simulink model "MX_Gateway"  (Simulink 9.0) (continued)
== stateflow chart MX_Gtwy_Control/System/Aux_Enable/Aux_Control == (continued)
          state Wait
            en:
            aux_Flow = AUX_RELEASE_WAIT;
            cmd_AuxRelease = INACTIVE;
          state Ack
            en:
            aux_Flow = AUX_RELEASE_ACK;
          state Release_Base
            en:
            aux_Flow = AUX_RELEASE_IN_PROGRESS;
            cmd_AuxRelease = BASE_ACTIVE;
          state Release_Rod
            en:
            cmd_AuxRelease = ROD_ACTIVE;
          state Confirm
            en:
            aux_Flow = AUX_RELEASE_CONFIRM;
            cmd_AuxRelease = INACTIVE;
          (default) -> Aux_Enable_State.PHOENIX_K2.Functional.Aux_Relief_G5.Wait
          Aux_Enable_State.PHOENIX_K2.Functional.Aux_Relief_G5.Wait -> Ack : [button_Aux]
          Ack -> Aux_Enable_State.PHOENIX_K2.Functional.Aux_Relief_G5.Wait [order 2] : [button_AuxReleaseBack]
          Aux_Enable_State.PHOENIX_K2.Functional.Aux_Relief_G5.Confirm -> Aux_Enable_State.PHOENIX_K2.Functional.Aux_Relief_G5.Wait : [after(CD_AUX_CONFIRM_TIME, tick)...
              | || button_AuxReleaseExit]
          Ack -> Aux_Enable_State.PHOENIX_K2.Functional.Aux_Relief_G5.Release_Base [order 1] : [button_AuxReleaseAck]
          Aux_Enable_State.PHOENIX_K2.Functional.Aux_Relief_G5.Release_Base -> Aux_Enable_State.PHOENIX_K2.Functional.Aux_Relief_G5.Release_Rod : [after(CD_AUX_RELEASE_TIME, tick)]
          Aux_Enable_State.PHOENIX_K2.Functional.Aux_Relief_G5.Release_Rod -> Aux_Enable_State.PHOENIX_K2.Functional.Aux_Relief_G5.Confirm : [after(CD_AUX_RELEASE_TIME, tick)]
        state Aux_Relief_G55 [commented out]
          state Wait [commented out]
            en:
            state_AuxRelease = ACTIVE;
            cmd_AuxRelease = INACTIVE;
          state Release_Base [commented out]
            en:
            cmd_AuxRelease = BASE_ACTIVE;
          state Release_Rod [commented out]
            en:
            cmd_AuxRelease = ROD_ACTIVE;
          state Confirm [commented out]
            en:
            cmd_AuxRelease = INACTIVE;
          (default) -> Aux_Enable_State.PHOENIX_K2.Functional.Aux_Relief_G55.Wait [commented out]
          Aux_Enable_State.PHOENIX_K2.Functional.Aux_Relief_G55.Wait -> Aux_Enable_State.PHOENIX_K2.Functional.Aux_Relief_G55.Release_Base [commented out] : [dlx_G55_AuxReleaseStatus == ACTIVE]
          Aux_Enable_State.PHOENIX_K2.Functional.Aux_Relief_G55.Confirm -> Aux_Enable_State.PHOENIX_K2.Functional.Aux_Relief_G55.Wait [commented out] : [dlx_G55_AuxReleaseStatus == INACTIVE]
          Aux_Enable_State.PHOENIX_K2.Functional.Aux_Relief_G55.Release_Base -> Aux_Enable_State.PHOENIX_K2.Functional.Aux_Relief_G55.Release_Rod [commented out] : [after(CD_AUX_RELEASE_TIME, tick)]
          Aux_Enable_State.PHOENIX_K2.Functional.Aux_Relief_G55.Release_Rod -> Aux_Enable_State.PHOENIX_K2.Functional.Aux_Relief_G55.Confirm [commented out] : [after(CD_AUX_RELEASE_TIME, tick)]
        (default) -> Aux_Enable_State.PHOENIX_K2.Functional.Inactive
        Aux_Enable_State.PHOENIX_K2.Functional.Inactive_Fault -> Aux_Enable_State.PHOENIX_K2.Functional.Enabled.Off : [state_AuxPot != OORH...
            |  && state_AuxPot != OORL...
            |  && state_System == SYS_NORMAL]
        Aux_Enable_State.PHOENIX_K2.Functional.Inactive -> Aux_Enable_State.PHOENIX_K2.Functional.Enabled [order 1] : [state_Engine != ENGINE_CRANKING...
            |  && (state_Engine == ENGINE_RUNNING...
            |     || CD_AUX_RELEASE_FUNCTION == NON_FUNCTIONAL...
            |     || (!ee_DeluxeG5...
            |          && CD_DELUXE_GEN5_5_FUNCTION == NON_FUNCTIONAL))...
            |  && state_System == SYS_NORMAL...
            |  && state_Console == ACTIVE...
            |  && flag_InitEERead]
        Aux_Enable_State.PHOENIX_K2.Functional.Enabled -> Aux_Enable_State.PHOENIX_K2.Functional.Inactive_Fault [order 2] : [state_AuxPot == OORH...
            |  || state_AuxPot == OORL]
        Aux_Enable_State.PHOENIX_K2.Functional.Enabled -> Aux_Enable_State.PHOENIX_K2.Functional.Inactive [order 1] : [state_Engine == ENGINE_CRANKING...
            |  || state_System != SYS_NORMAL...
            |  || state_Console != ACTIVE]
        Aux_Enable_State.PHOENIX_K2.Functional.Inactive_Out_Of_Neutral -> Aux_Enable_State.PHOENIX_K2.Functional.Inactive_Fault [order 2] : [state_AuxPot == OORH...
            |  || state_AuxPot == OORL]
        Aux_Enable_State.PHOENIX_K2.Functional.Enabled -> Aux_Enable_State.PHOENIX_K2.Functional.Inactive_Out_Of_Neutral [order 3] : [state_AuxPot == NOT_IN_NEUTRAL]
        (junction 2117) -> Aux_Enable_State.PHOENIX_K2.Functional.Enabled
        (junction 2116) -> (junction 2115) [order 1] : [(aux_DebounceButton...
            | || aux_DetentHold)...
            | && aux_Flow != AUX_FLOW_INACTIVE]
        Aux_Enable_State.PHOENIX_K2.Functional.Inactive_Out_Of_Neutral -> (junction 2116) [order 3] : [state_AuxPot != NOT_IN_NEUTRAL]
        (junction 2115) -> (junction 2117) : {state_Buzzer = SINGLE_SHORT_BEEP;}
        (junction 2116) -> (junction 2118) [order 2]
        (junction 2118) -> (junction 2117)
        Aux_Enable_State.PHOENIX_K2.Functional.Inactive_Out_Of_Neutral -> Aux_Enable_State.PHOENIX_K2.Functional.Inactive [order 1] : [state_Console != ACTIVE]
        Aux_Enable_State.PHOENIX_K2.Functional.Inactive -> Aux_Relief_G5 [order 2] : [(CD_AUX_RELEASE_FUNCTION != NON_FUNCTIONAL)...
            | && ((state_Engine == ENGINE_NOT_RUNNING) || (state_Engine == ENGINE_INITIALIZE))...
            | && (state_EngineSpeed != NO_SIGNAL)...
            | && (state_System == SYS_NORMAL)...
            | && (ee_DeluxeG5) && flag_InitEERead...
            | && (CD_DELUXE_GEN5_5_FUNCTION == NON_FUNCTIONAL)]
        Aux_Relief_G5 -> Aux_Enable_State.PHOENIX_K2.Functional.Inactive : [((state_Engine != ENGINE_NOT_RUNNING) && (state_Engine != ENGINE_INITIALIZE))...
            |  || (state_EngineSpeed == NO_SIGNAL)...
            |  || (state_System != SYS_NORMAL)]
        Aux_Enable_State.PHOENIX_K2.Functional.Inactive -> Aux_Relief_G55 [commented out] : [(CD_AUX_RELEASE_FUNCTION != NON_FUNCTIONAL)...
            | && ((state_Engine == ENGINE_NOT_RUNNING) || (state_Engine == ENGINE_INITIALIZE))...
            | && (state_EngineSpeed != NO_SIGNAL)...
            | && (state_System == SYS_NORMAL)...
            | && (!ee_DeluxeG5)...
            | && (CD_DELUXE_GEN5_5_FUNCTION != NON_FUNCTIONAL)]
        Aux_Relief_G55 -> Aux_Enable_State.PHOENIX_K2.Functional.Inactive [commented out] : [((state_Engine != ENGINE_NOT_RUNNING) && (state_Engine != ENGINE_INITIALIZE))...
            |  || (state_System != SYS_NORMAL)]
      state Aux_Enable_State_ST
        state Inactive_Latched
          en:
          state_AuxEnable = INACTIVE;
        state Inactive_Temp
          en:
          state_AuxEnable = INACTIVE;
        state Standard_Flow
          en:
          aux_Flow = AUX_FLOW_STANDARD;
          state_AuxEnable = ACTIVE_MODE_ONE;
          ee_AuxFlow = AUX_FLOW_INACTIVE;
          matlab_UpdateEEVar();
        (default) -> Aux_Enable_State.PHOENIX_K2.Aux_Enable_State_ST.Standard_Flow
        Aux_Enable_State.PHOENIX_K2.Aux_Enable_State_ST.Standard_Flow -> Aux_Enable_State.PHOENIX_K2.Aux_Enable_State_ST.Inactive_Temp [order 1] : [state_Engine == ENGINE_CRANKING]
        Aux_Enable_State.PHOENIX_K2.Aux_Enable_State_ST.Standard_Flow -> Aux_Enable_State.PHOENIX_K2.Aux_Enable_State_ST.Inactive_Latched [order 2] : [state_AuxPot == OORH...
            |  || state_AuxPot == OORL...
            |  || state_Console == OORL...
            |  || state_Console == OORH...
            |  || state_AuxPot == NOT_IN_NEUTRAL...
            |  || state_System != SYS_NORMAL]
        Aux_Enable_State.PHOENIX_K2.Aux_Enable_State_ST.Inactive_Temp -> Aux_Enable_State.PHOENIX_K2.Aux_Enable_State_ST.Standard_Flow : [state_Engine != ENGINE_CRANKING]
        Aux_Enable_State.PHOENIX_K2.Aux_Enable_State_ST.Standard_Flow -> Aux_Enable_State.PHOENIX_K2.Aux_Enable_State_ST.Inactive_Latched [order 3] : disable_aux
      state Aux_Enable_State_ACD
        state Inactive_Latched
          en:
          state_AuxEnable = INACTIVE;
        state Inactive_Temp
          en:
          state_AuxEnable = INACTIVE;
        state Standard_Flow
          en:
          aux_Flow = AUX_FLOW_STANDARD;
          state_AuxEnable = ACTIVE_MODE_ONE;
          ee_AuxFlow = AUX_FLOW_INACTIVE;
          matlab_UpdateEEVar();
        (default) -> Aux_Enable_State.PHOENIX_K2.Aux_Enable_State_ACD.Standard_Flow
        Aux_Enable_State.PHOENIX_K2.Aux_Enable_State_ACD.Standard_Flow -> Aux_Enable_State.PHOENIX_K2.Aux_Enable_State_ACD.Inactive_Temp [order 1] : [state_Engine == ENGINE_CRANKING]
        Aux_Enable_State.PHOENIX_K2.Aux_Enable_State_ACD.Standard_Flow -> Aux_Enable_State.PHOENIX_K2.Aux_Enable_State_ACD.Inactive_Latched [order 2] : [state_AuxPot == OORH...
            |  || state_AuxPot == OORL...
            |  || state_Console == OORL...
            |  || state_Console == OORH...
            |  || state_AuxPot == NOT_IN_NEUTRAL...
            |  || state_System != SYS_NORMAL]
        Aux_Enable_State.PHOENIX_K2.Aux_Enable_State_ACD.Standard_Flow -> Aux_Enable_State.PHOENIX_K2.Aux_Enable_State_ACD.Inactive_Latched [order 3] : disable_aux
        Aux_Enable_State.PHOENIX_K2.Aux_Enable_State_ACD.Inactive_Temp -> Aux_Enable_State.PHOENIX_K2.Aux_Enable_State_ACD.Standard_Flow : [state_Engine != ENGINE_CRANKING]
      (default) -> Aux_Enable_State.PHOENIX_K2.Functional
      Aux_Enable_State.PHOENIX_K2.Functional -> Aux_Enable_State.PHOENIX_K2.Aux_Enable_State_ST [order 1] : [(can_AuxEnable_ST == ACTIVE)...
          | && (state_ST_CAN == NORMAL)...
          | && (CD_STAUX_FUNCTION != NON_FUNCTIONAL)]
      Aux_Enable_State.PHOENIX_K2.Aux_Enable_State_ST -> Aux_Enable_State.PHOENIX_K2.Functional : [(can_AuxEnable_ST == INACTIVE)...
          | || (state_ST_CAN != NORMAL)]
      Aux_Enable_State.PHOENIX_K2.Functional -> Aux_Enable_State.PHOENIX_K2.Aux_Enable_State_ACD [order 2] : [(can_AuxEnable_ACD == ACTIVE)...
          | && (state_ACD_CAN == NORMAL)...
          | && (CD_RACD_FUNCTION != NON_FUNCTIONAL)]
      Aux_Enable_State.PHOENIX_K2.Aux_Enable_State_ACD -> Aux_Enable_State.PHOENIX_K2.Functional : [(can_AuxEnable_ACD == INACTIVE)...
          | || (state_ACD_CAN != NORMAL)]
    state SIRIUS2
      state Functional
        state Enabled
          state Off
            en:
            aux_Flow = AUX_FLOW_INACTIVE;
            ee_AuxFlow = AUX_FLOW_INACTIVE;
            state_AuxEnable = INACTIVE;
            matlab_UpdateEEVar();
          state Active
            state Low_Flow
              en:
              aux_Flow = AUX_FLOW_LOW;
              ee_AuxFlow = AUX_FLOW_LOW;
              state_AuxEnable = ACTIVE_MODE_ONE;
              matlab_UpdateEEVar();
            state Medium_Flow
              en:
              aux_Flow = AUX_FLOW_MEDIUM;
              ee_AuxFlow = AUX_FLOW_MEDIUM;
              state_AuxEnable = ACTIVE_MODE_ONE;
              matlab_UpdateEEVar();
            state Standard_Flow
              en:
              aux_Flow = AUX_FLOW_STANDARD;
              ee_AuxFlow = AUX_FLOW_STANDARD;
              state_AuxEnable = ACTIVE_MODE_ONE;
              matlab_UpdateEEVar();
            Aux_Enable_State.SIRIUS2.Functional.Enabled.Active.Medium_Flow -> Aux_Enable_State.SIRIUS2.Functional.Enabled.Active.Low_Flow : [aux_DebounceButton]
                | {state_Buzzer = SINGLE_SHORT_BEEP;}
            Aux_Enable_State.SIRIUS2.Functional.Enabled.Active.Low_Flow -> Aux_Enable_State.SIRIUS2.Functional.Enabled.Active.Standard_Flow [order 1] : [aux_DebounceButton...
                | && state_DetentEnable == ACTIVE]
                | {state_Buzzer = SINGLE_SHORT_BEEP;}
            Aux_Enable_State.SIRIUS2.Functional.Enabled.Active.Standard_Flow -> Aux_Enable_State.SIRIUS2.Functional.Enabled.Active.Medium_Flow : [aux_DebounceButton]
                | {state_Buzzer = SINGLE_SHORT_BEEP;}
          (junction 2206) -> Aux_Enable_State.SIRIUS2.Functional.Enabled.Off [order 1] : [ee_AuxFlow == AUX_FLOW_INACTIVE]
          (default) -> (junction 2206)
          (junction 2206) -> (junction 2207) [order 2]
          Aux_Enable_State.SIRIUS2.Functional.Enabled.Active.Low_Flow -> Aux_Enable_State.SIRIUS2.Functional.Enabled.Off [order 2] : [aux_DebounceButton...
              | && state_DetentEnable != ACTIVE]
              | {state_Buzzer = SINGLE_SHORT_BEEP;}
          Aux_Enable_State.SIRIUS2.Functional.Enabled.Off -> Aux_Enable_State.SIRIUS2.Functional.Enabled.Active.Standard_Flow : [aux_DebounceButton...
              | || aux_DetentHold]
              | {state_Buzzer = SINGLE_SHORT_BEEP;}
          Aux_Enable_State.SIRIUS2.Functional.Enabled.Active -> Aux_Enable_State.SIRIUS2.Functional.Enabled.Off : disable_aux
          (junction 2207) -> Aux_Enable_State.SIRIUS2.Functional.Enabled.Active.Low_Flow [order 1] : [ee_AuxFlow == AUX_FLOW_LOW]
          (junction 2207) -> (junction 2208) [order 2]
          (junction 2208) -> Aux_Enable_State.SIRIUS2.Functional.Enabled.Active.Medium_Flow [order 1] : [ee_AuxFlow == AUX_FLOW_MEDIUM]
          (junction 2208) -> (junction 2209) [order 2]
          (junction 2209) -> Aux_Enable_State.SIRIUS2.Functional.Enabled.Active.Standard_Flow
        state Inactive
          en:
          state_AuxEnable = INACTIVE;
          cmd_AuxRelease = INACTIVE;
          aux_Flow = AUX_FLOW_INACTIVE;
        state Inactive_Fault
          en:
          state_AuxEnable = INACTIVE;
          aux_Flow = AUX_FLOW_INACTIVE;
        state Inactive_Out_Of_Neutral
          en:
          state_AuxEnable = INACTIVE;
          aux_Flow = AUX_FLOW_INACTIVE;
        (default) -> Aux_Enable_State.SIRIUS2.Functional.Inactive
        Aux_Enable_State.SIRIUS2.Functional.Inactive_Fault -> Aux_Enable_State.SIRIUS2.Functional.Enabled.Off : [state_AuxPot != OORH...
            |  && state_AuxPot != OORL...
            |  && state_System == SYS_NORMAL]
        Aux_Enable_State.SIRIUS2.Functional.Inactive -> Aux_Enable_State.SIRIUS2.Functional.Enabled : [state_Engine != ENGINE_CRANKING...
            |  && state_System == SYS_NORMAL...
            |  && state_Console == ACTIVE...
            |  && flag_InitEERead]
        Aux_Enable_State.SIRIUS2.Functional.Enabled -> Aux_Enable_State.SIRIUS2.Functional.Inactive_Fault [order 2] : [state_AuxPot == OORH...
            |  || state_AuxPot == OORL]
        Aux_Enable_State.SIRIUS2.Functional.Enabled -> Aux_Enable_State.SIRIUS2.Functional.Inactive [order 1] : [state_Engine == ENGINE_CRANKING...
            |  || state_System != SYS_NORMAL...
            |  || state_Console != ACTIVE]
        Aux_Enable_State.SIRIUS2.Functional.Inactive_Out_Of_Neutral -> Aux_Enable_State.SIRIUS2.Functional.Inactive_Fault [order 2] : [state_AuxPot == OORH...
            |  || state_AuxPot == OORL]
        Aux_Enable_State.SIRIUS2.Functional.Enabled -> Aux_Enable_State.SIRIUS2.Functional.Inactive_Out_Of_Neutral [order 3] : [state_AuxPot == NOT_IN_NEUTRAL]
        (junction 2190) -> Aux_Enable_State.SIRIUS2.Functional.Enabled
        (junction 2189) -> (junction 2188) [order 1] : [(aux_DebounceButton...
            | || aux_DetentHold)...
            | && aux_Flow != AUX_FLOW_INACTIVE]
        Aux_Enable_State.SIRIUS2.Functional.Inactive_Out_Of_Neutral -> (junction 2189) [order 3] : [state_AuxPot != NOT_IN_NEUTRAL]
        (junction 2188) -> (junction 2190) : {state_Buzzer = SINGLE_SHORT_BEEP;}
        (junction 2189) -> (junction 2191) [order 2]
        (junction 2191) -> (junction 2190)
        Aux_Enable_State.SIRIUS2.Functional.Inactive_Out_Of_Neutral -> Aux_Enable_State.SIRIUS2.Functional.Inactive [order 1] : [state_Console != ACTIVE]
      state Aux_Enable_State_ST
        state Inactive_Latched
          en:
          state_AuxEnable = INACTIVE;
        state Inactive_Temp
          en:
          state_AuxEnable = INACTIVE;
        state Standard_Flow
          en:
          aux_Flow = AUX_FLOW_STANDARD;
          state_AuxEnable = ACTIVE_MODE_ONE;
          ee_AuxFlow = AUX_FLOW_INACTIVE;
          matlab_UpdateEEVar();
        (default) -> Aux_Enable_State.SIRIUS2.Aux_Enable_State_ST.Standard_Flow
        Aux_Enable_State.SIRIUS2.Aux_Enable_State_ST.Standard_Flow -> Aux_Enable_State.SIRIUS2.Aux_Enable_State_ST.Inactive_Temp [order 1] : [state_Engine == ENGINE_CRANKING]
        Aux_Enable_State.SIRIUS2.Aux_Enable_State_ST.Standard_Flow -> Aux_Enable_State.SIRIUS2.Aux_Enable_State_ST.Inactive_Latched [order 2] : [state_AuxPot == OORH...
            |  || state_AuxPot == OORL...
            |  || state_Console == OORL...
            |  || state_Console == OORH...
            |  || state_AuxPot == NOT_IN_NEUTRAL...
            |  || state_System != SYS_NORMAL]
        Aux_Enable_State.SIRIUS2.Aux_Enable_State_ST.Inactive_Temp -> Aux_Enable_State.SIRIUS2.Aux_Enable_State_ST.Standard_Flow : [state_Engine != ENGINE_CRANKING]
        Aux_Enable_State.SIRIUS2.Aux_Enable_State_ST.Standard_Flow -> Aux_Enable_State.SIRIUS2.Aux_Enable_State_ST.Inactive_Latched [order 3] : disable_aux
      state Aux_Enable_State_ACD
        state Inactive_Latched
          en:
          state_AuxEnable = INACTIVE;
        state Inactive_Temp
          en:
          state_AuxEnable = INACTIVE;
        state Standard_Flow
          en:
          aux_Flow = AUX_FLOW_STANDARD;
          state_AuxEnable = ACTIVE_MODE_ONE;
          ee_AuxFlow = AUX_FLOW_INACTIVE;
          matlab_UpdateEEVar();
        (default) -> Aux_Enable_State.SIRIUS2.Aux_Enable_State_ACD.Standard_Flow
        Aux_Enable_State.SIRIUS2.Aux_Enable_State_ACD.Standard_Flow -> Aux_Enable_State.SIRIUS2.Aux_Enable_State_ACD.Inactive_Temp [order 1] : [state_Engine == ENGINE_CRANKING]
        Aux_Enable_State.SIRIUS2.Aux_Enable_State_ACD.Standard_Flow -> Aux_Enable_State.SIRIUS2.Aux_Enable_State_ACD.Inactive_Latched [order 2] : [state_AuxPot == OORH...
            |  || state_AuxPot == OORL...
            |  || state_Console == OORL...
            |  || state_Console == OORH...
            |  || state_AuxPot == NOT_IN_NEUTRAL...
            |  || state_System != SYS_NORMAL]
        Aux_Enable_State.SIRIUS2.Aux_Enable_State_ACD.Standard_Flow -> Aux_Enable_State.SIRIUS2.Aux_Enable_State_ACD.Inactive_Latched [order 3] : disable_aux
        Aux_Enable_State.SIRIUS2.Aux_Enable_State_ACD.Inactive_Temp -> Aux_Enable_State.SIRIUS2.Aux_Enable_State_ACD.Standard_Flow : [state_Engine != ENGINE_CRANKING]
      (default) -> Aux_Enable_State.SIRIUS2.Functional
      Aux_Enable_State.SIRIUS2.Functional -> Aux_Enable_State.SIRIUS2.Aux_Enable_State_ST [order 1] : [(can_AuxEnable_ST == ACTIVE)...
          | && (state_ST_CAN == NORMAL)...
          | && (CD_STAUX_FUNCTION != NON_FUNCTIONAL)]
      Aux_Enable_State.SIRIUS2.Aux_Enable_State_ST -> Aux_Enable_State.SIRIUS2.Functional : [(can_AuxEnable_ST == INACTIVE)...
          | || (state_ST_CAN != NORMAL)]
      Aux_Enable_State.SIRIUS2.Functional -> Aux_Enable_State.SIRIUS2.Aux_Enable_State_ACD [order 2] : [(can_AuxEnable_ACD == ACTIVE)...
          | && (state_ACD_CAN == NORMAL)...
          | && (CD_RACD_FUNCTION != NON_FUNCTIONAL)]
      Aux_Enable_State.SIRIUS2.Aux_Enable_State_ACD -> Aux_Enable_State.SIRIUS2.Functional : [(can_AuxEnable_ACD == INACTIVE)...
          | || (state_ACD_CAN != NORMAL)]
    state SIRIUS2_HIFLOW
      state Functional
        state Enabled
          state Off
            en:
            aux_Flow = AUX_FLOW_INACTIVE;
            ee_AuxFlow = AUX_FLOW_INACTIVE;
            state_AuxEnable = INACTIVE;
            matlab_UpdateEEVar();
          state Active
            state Low_Flow
              en:
              aux_Flow = AUX_FLOW_LOW;
              ee_AuxFlow = AUX_FLOW_LOW;
              state_AuxEnable = ACTIVE_MODE_ONE;
              matlab_UpdateEEVar();
            state Medium_Flow
              en:
              aux_Flow = AUX_FLOW_MEDIUM;
              ee_AuxFlow = AUX_FLOW_MEDIUM;
              state_AuxEnable = ACTIVE_MODE_ONE;
              matlab_UpdateEEVar();
            state Standard_Flow
              en:
              aux_Flow = AUX_FLOW_STANDARD;
              ee_AuxFlow = AUX_FLOW_STANDARD;
              state_AuxEnable = ACTIVE_MODE_ONE;
              matlab_UpdateEEVar();
            state High_Flow
              en:
              aux_Flow = AUX_FLOW_HIGH;
              ee_AuxFlow = AUX_FLOW_HIGH;
              state_AuxEnable = ACTIVE_MODE_ONE;
              matlab_UpdateEEVar();
            Aux_Enable_State.SIRIUS2_HIFLOW.Functional.Enabled.Active.Medium_Flow -> Aux_Enable_State.SIRIUS2_HIFLOW.Functional.Enabled.Active.Low_Flow : [aux_DebounceButton]
                | {state_Buzzer = SINGLE_SHORT_BEEP;}
            Aux_Enable_State.SIRIUS2_HIFLOW.Functional.Enabled.Active.Low_Flow -> High_Flow [order 1] : [aux_DebounceButton...
                | && state_DetentEnable == ACTIVE]
                | {state_Buzzer = SINGLE_SHORT_BEEP;}
            Aux_Enable_State.SIRIUS2_HIFLOW.Functional.Enabled.Active.Standard_Flow -> Aux_Enable_State.SIRIUS2_HIFLOW.Functional.Enabled.Active.Medium_Flow : [aux_DebounceButton]
                | {state_Buzzer = SINGLE_SHORT_BEEP;}
            High_Flow -> Aux_Enable_State.SIRIUS2_HIFLOW.Functional.Enabled.Active.Standard_Flow : [aux_DebounceButton]
                | {state_Buzzer = SINGLE_SHORT_BEEP;}
          (junction 2257) -> Aux_Enable_State.SIRIUS2_HIFLOW.Functional.Enabled.Off [order 1] : [ee_AuxFlow == AUX_FLOW_INACTIVE]
          (default) -> (junction 2257)
          (junction 2257) -> (junction 2258) [order 2]
          Aux_Enable_State.SIRIUS2_HIFLOW.Functional.Enabled.Active.Low_Flow -> Aux_Enable_State.SIRIUS2_HIFLOW.Functional.Enabled.Off [order 2] : [aux_DebounceButton...
              | && state_DetentEnable != ACTIVE]
              | {state_Buzzer = SINGLE_SHORT_BEEP;}
          Aux_Enable_State.SIRIUS2_HIFLOW.Functional.Enabled.Off -> High_Flow : [aux_DebounceButton...
              | || aux_DetentHold]
              | {state_Buzzer = SINGLE_SHORT_BEEP;}
          Aux_Enable_State.SIRIUS2_HIFLOW.Functional.Enabled.Active -> Aux_Enable_State.SIRIUS2_HIFLOW.Functional.Enabled.Off : disable_aux
          (junction 2258) -> Aux_Enable_State.SIRIUS2_HIFLOW.Functional.Enabled.Active.Low_Flow [order 1] : [ee_AuxFlow == AUX_FLOW_LOW]
          (junction 2258) -> (junction 2259) [order 2]
          (junction 2259) -> Aux_Enable_State.SIRIUS2_HIFLOW.Functional.Enabled.Active.Medium_Flow [order 1] : [ee_AuxFlow == AUX_FLOW_MEDIUM]
          (junction 2259) -> (junction 2260) [order 2]
          (junction 2260) -> Aux_Enable_State.SIRIUS2_HIFLOW.Functional.Enabled.Active.Standard_Flow [order 1] : [ee_AuxFlow == AUX_FLOW_STANDARD]
          (junction 2260) -> (junction 2261) [order 2]
          (junction 2261) -> High_Flow
        state Inactive
          en:
          state_AuxEnable = INACTIVE;
          cmd_AuxRelease = INACTIVE;
          aux_Flow = AUX_FLOW_INACTIVE;
        state Inactive_Fault
          en:
          state_AuxEnable = INACTIVE;
          aux_Flow = AUX_FLOW_INACTIVE;
        state Inactive_Out_Of_Neutral
          en:
          state_AuxEnable = INACTIVE;
          aux_Flow = AUX_FLOW_INACTIVE;
        (default) -> Aux_Enable_State.SIRIUS2_HIFLOW.Functional.Inactive
        Aux_Enable_State.SIRIUS2_HIFLOW.Functional.Inactive_Fault -> Aux_Enable_State.SIRIUS2_HIFLOW.Functional.Enabled.Off : [state_AuxPot != OORH...
            |  && state_AuxPot != OORL...
            |  && state_System == SYS_NORMAL]
        Aux_Enable_State.SIRIUS2_HIFLOW.Functional.Inactive -> Aux_Enable_State.SIRIUS2_HIFLOW.Functional.Enabled : [state_Engine != ENGINE_CRANKING...
            |  && state_System == SYS_NORMAL...
            |  && state_Console == ACTIVE...
            |  && flag_InitEERead]
        Aux_Enable_State.SIRIUS2_HIFLOW.Functional.Enabled -> Aux_Enable_State.SIRIUS2_HIFLOW.Functional.Inactive_Fault [order 2] : [state_AuxPot == OORH...
            |  || state_AuxPot == OORL]
        Aux_Enable_State.SIRIUS2_HIFLOW.Functional.Enabled -> Aux_Enable_State.SIRIUS2_HIFLOW.Functional.Inactive [order 1] : [state_Engine == ENGINE_CRANKING...
            |  || state_System != SYS_NORMAL...
            |  || state_Console != ACTIVE]
        Aux_Enable_State.SIRIUS2_HIFLOW.Functional.Inactive_Out_Of_Neutral -> Aux_Enable_State.SIRIUS2_HIFLOW.Functional.Inactive_Fault [order 2] : [state_AuxPot == OORH...
            |  || state_AuxPot == OORL]
        Aux_Enable_State.SIRIUS2_HIFLOW.Functional.Enabled -> Aux_Enable_State.SIRIUS2_HIFLOW.Functional.Inactive_Out_Of_Neutral [order 3] : [state_AuxPot == NOT_IN_NEUTRAL]
        (junction 2241) -> Aux_Enable_State.SIRIUS2_HIFLOW.Functional.Enabled
        (junction 2240) -> (junction 2239) [order 1] : [(aux_DebounceButton...
            | || aux_DetentHold)...
            | && aux_Flow != AUX_FLOW_INACTIVE]
        Aux_Enable_State.SIRIUS2_HIFLOW.Functional.Inactive_Out_Of_Neutral -> (junction 2240) [order 3] : [state_AuxPot != NOT_IN_NEUTRAL]
        (junction 2239) -> (junction 2241) : {state_Buzzer = SINGLE_SHORT_BEEP;}
        (junction 2240) -> (junction 2242) [order 2]
        (junction 2242) -> (junction 2241)
        Aux_Enable_State.SIRIUS2_HIFLOW.Functional.Inactive_Out_Of_Neutral -> Aux_Enable_State.SIRIUS2_HIFLOW.Functional.Inactive [order 1] : [state_Console != ACTIVE]
      state Aux_Enable_State_ST
        state Inactive_Latched
          en:
          state_AuxEnable = INACTIVE;
        state Inactive_Temp
          en:
          state_AuxEnable = INACTIVE;
        state Standard_Flow
          en:
          aux_Flow = AUX_FLOW_STANDARD;
          state_AuxEnable = ACTIVE_MODE_ONE;
          ee_AuxFlow = AUX_FLOW_INACTIVE;
          matlab_UpdateEEVar();
        (default) -> Aux_Enable_State.SIRIUS2_HIFLOW.Aux_Enable_State_ST.Standard_Flow
        Aux_Enable_State.SIRIUS2_HIFLOW.Aux_Enable_State_ST.Standard_Flow -> Aux_Enable_State.SIRIUS2_HIFLOW.Aux_Enable_State_ST.Inactive_Temp [order 1] : [state_Engine == ENGINE_CRANKING]
        Aux_Enable_State.SIRIUS2_HIFLOW.Aux_Enable_State_ST.Standard_Flow -> Aux_Enable_State.SIRIUS2_HIFLOW.Aux_Enable_State_ST.Inactive_Latched [order 2] : [state_AuxPot == OORH...
            |  || state_AuxPot == OORL...
            |  || state_Console == OORL...
            |  || state_Console == OORH...
            |  || state_AuxPot == NOT_IN_NEUTRAL...
            |  || state_System != SYS_NORMAL]
        Aux_Enable_State.SIRIUS2_HIFLOW.Aux_Enable_State_ST.Inactive_Temp -> Aux_Enable_State.SIRIUS2_HIFLOW.Aux_Enable_State_ST.Standard_Flow : [state_Engine != ENGINE_CRANKING]
        Aux_Enable_State.SIRIUS2_HIFLOW.Aux_Enable_State_ST.Standard_Flow -> Aux_Enable_State.SIRIUS2_HIFLOW.Aux_Enable_State_ST.Inactive_Latched [order 3] : disable_aux
      state Aux_Enable_State_ACD
        state Inactive_Latched
          en:
          state_AuxEnable = INACTIVE;
        state Inactive_Temp
          en:
          state_AuxEnable = INACTIVE;
        state Standard_Flow
          en:
          aux_Flow = AUX_FLOW_STANDARD;
          state_AuxEnable = ACTIVE_MODE_ONE;
          ee_AuxFlow = AUX_FLOW_INACTIVE;
          matlab_UpdateEEVar();
        (default) -> Aux_Enable_State.SIRIUS2_HIFLOW.Aux_Enable_State_ACD.Standard_Flow
        Aux_Enable_State.SIRIUS2_HIFLOW.Aux_Enable_State_ACD.Standard_Flow -> Aux_Enable_State.SIRIUS2_HIFLOW.Aux_Enable_State_ACD.Inactive_Temp [order 1] : [state_Engine == ENGINE_CRANKING]
        Aux_Enable_State.SIRIUS2_HIFLOW.Aux_Enable_State_ACD.Standard_Flow -> Aux_Enable_State.SIRIUS2_HIFLOW.Aux_Enable_State_ACD.Inactive_Latched [order 2] : [state_AuxPot == OORH...
            |  || state_AuxPot == OORL...
            |  || state_Console == OORL...
            |  || state_Console == OORH...
            |  || state_AuxPot == NOT_IN_NEUTRAL...
            |  || state_System != SYS_NORMAL]
        Aux_Enable_State.SIRIUS2_HIFLOW.Aux_Enable_State_ACD.Standard_Flow -> Aux_Enable_State.SIRIUS2_HIFLOW.Aux_Enable_State_ACD.Inactive_Latched [order 3] : disable_aux
        Aux_Enable_State.SIRIUS2_HIFLOW.Aux_Enable_State_ACD.Inactive_Temp -> Aux_Enable_State.SIRIUS2_HIFLOW.Aux_Enable_State_ACD.Standard_Flow : [state_Engine != ENGINE_CRANKING]
      (default) -> Aux_Enable_State.SIRIUS2_HIFLOW.Functional
      Aux_Enable_State.SIRIUS2_HIFLOW.Functional -> Aux_Enable_State.SIRIUS2_HIFLOW.Aux_Enable_State_ST [order 1] : [(can_AuxEnable_ST == ACTIVE)...
          | && (state_ST_CAN == NORMAL)...
          | && (CD_STAUX_FUNCTION != NON_FUNCTIONAL)]
      Aux_Enable_State.SIRIUS2_HIFLOW.Aux_Enable_State_ST -> Aux_Enable_State.SIRIUS2_HIFLOW.Functional : [(can_AuxEnable_ST == INACTIVE)...
          | || (state_ST_CAN != NORMAL)]
      Aux_Enable_State.SIRIUS2_HIFLOW.Functional -> Aux_Enable_State.SIRIUS2_HIFLOW.Aux_Enable_State_ACD [order 2] : [(can_AuxEnable_ACD == ACTIVE)...
          | && (state_ACD_CAN == NORMAL)...
          | && (CD_RACD_FUNCTION != NON_FUNCTIONAL)]
      Aux_Enable_State.SIRIUS2_HIFLOW.Aux_Enable_State_ACD -> Aux_Enable_State.SIRIUS2_HIFLOW.Functional : [(can_AuxEnable_ACD == INACTIVE)...
          | || (state_ACD_CAN != NORMAL)]
    state EMEA_CONTROLS
      state Functional
        en:
        previouslyActive = 0;
        state Enabled
          state Off
            en:
            aux_Flow = AUX_FLOW_INACTIVE;
            state_AuxEnable = INACTIVE;
            ee_AuxFlow = AUX_FLOW_INACTIVE;
            matlab_UpdateEEVar();
          state WAIT_FOR_AUX_PRESS
          state Active
            en:
            previouslyActive = 1;
            state Low_Flow
              en:
              aux_Flow = AUX_FLOW_LOW;
              state_AuxEnable = ACTIVE_MODE_ONE;
              ee_AuxFlow = AUX_FLOW_LOW;
              matlab_UpdateEEVar();
            state Medium_Flow
              en:
              aux_Flow = AUX_FLOW_MEDIUM;
              state_AuxEnable = ACTIVE_MODE_ONE;
              ee_AuxFlow = AUX_FLOW_MEDIUM;
              matlab_UpdateEEVar();
            state Standard_Flow
              en:
              aux_Flow = AUX_FLOW_STANDARD;
              state_AuxEnable = ACTIVE_MODE_ONE;
              ee_AuxFlow = AUX_FLOW_STANDARD;
              matlab_UpdateEEVar();
            Aux_Enable_State.EMEA_CONTROLS.Functional.Enabled.Active.Medium_Flow -> Aux_Enable_State.EMEA_CONTROLS.Functional.Enabled.Active.Low_Flow : [aux_DebounceButton]
                | {state_Buzzer = SINGLE_SHORT_BEEP;}
            Aux_Enable_State.EMEA_CONTROLS.Functional.Enabled.Active.Low_Flow -> Aux_Enable_State.EMEA_CONTROLS.Functional.Enabled.Active.Standard_Flow [order 1] : [aux_DebounceButton...
                | && state_DetentEnable == ACTIVE]
                | {state_Buzzer = SINGLE_SHORT_BEEP;}
            Aux_Enable_State.EMEA_CONTROLS.Functional.Enabled.Active.Standard_Flow -> Aux_Enable_State.EMEA_CONTROLS.Functional.Enabled.Active.Medium_Flow : [aux_DebounceButton]
                | {state_Buzzer = SINGLE_SHORT_BEEP;}
          (default) -> (junction 2306)
          (junction 2306) -> Aux_Enable_State.EMEA_CONTROLS.Functional.Enabled.Off [order 1] : [ee_AuxFlow == AUX_FLOW_INACTIVE]
          (junction 2306) -> (junction 2307) [order 2]
          (junction 2307) -> WAIT_FOR_AUX_PRESS [order 2]
          Aux_Enable_State.EMEA_CONTROLS.Functional.Enabled.Active.Low_Flow -> Aux_Enable_State.EMEA_CONTROLS.Functional.Enabled.Off [order 2] : [aux_DebounceButton...
              | && state_DetentEnable != ACTIVE]
              | {state_Buzzer = SINGLE_SHORT_BEEP;}
          Aux_Enable_State.EMEA_CONTROLS.Functional.Enabled.Active -> Aux_Enable_State.EMEA_CONTROLS.Functional.Enabled.Off [order 1] : disable_aux
          Aux_Enable_State.EMEA_CONTROLS.Functional.Enabled.Active -> Aux_Enable_State.EMEA_CONTROLS.Functional.Enabled.Off [order 2] : [change_OffsetSelectSw...
              | || aux_Inhibit]
          Aux_Enable_State.EMEA_CONTROLS.Functional.Enabled.Off -> Aux_Enable_State.EMEA_CONTROLS.Functional.Enabled.Active.Standard_Flow : [!aux_Inhibit...
              | &&(aux_DebounceButton || aux_DetentHold)]
              | {state_Buzzer = SINGLE_SHORT_BEEP;}
          Aux_Enable_State.EMEA_CONTROLS.Functional.Enabled.Active -> Aux_Enable_State.EMEA_CONTROLS.Functional.Enabled.Off [order 3] : [aux_DebounceButton...
              | && mode_RightControl != MODE_RIGHT_AUX1]
              | {state_Buzzer = SINGLE_SHORT_BEEP;}
          (junction 2307) -> (junction 2308) [order 1] : [((CD_EMEA_CONTROLS_OPTION_B == NON_FUNCTIONAL)...
              | && (CD_EMEA_CONTROLS_OPTION_C == NON_FUNCTIONAL))...
              | || previouslyActive]
          WAIT_FOR_AUX_PRESS -> (junction 2308) : [!aux_Inhibit...
              | &&(aux_DebounceButton || aux_DetentHold)]
              | {state_Buzzer = SINGLE_SHORT_BEEP;}
          (junction 2308) -> (junction 2309)
          (junction 2309) -> Aux_Enable_State.EMEA_CONTROLS.Functional.Enabled.Active.Low_Flow [order 1] : [ee_AuxFlow == AUX_FLOW_LOW...
              | && !ee_DeluxeG5...
              | && CD_AUX_ADJ_FLOW_FUNCTION != NON_FUNCTIONAL]
          (junction 2309) -> (junction 2310) [order 2]
          (junction 2310) -> Aux_Enable_State.EMEA_CONTROLS.Functional.Enabled.Active.Medium_Flow [order 1] : [ee_AuxFlow == AUX_FLOW_MEDIUM...
              | && !ee_DeluxeG5...
              | && CD_AUX_ADJ_FLOW_FUNCTION != NON_FUNCTIONAL]
          (junction 2310) -> (junction 2311) [order 2]
          (junction 2311) -> Aux_Enable_State.EMEA_CONTROLS.Functional.Enabled.Active.Standard_Flow
        state Inactive
          en:
          state_AuxEnable = INACTIVE;
          cmd_AuxRelease = INACTIVE;
          aux_Flow = AUX_FLOW_INACTIVE;
          state_AuxRelease = INACTIVE;
        state Inactive_Fault
          en:
          state_AuxEnable = INACTIVE;
          aux_Flow = AUX_FLOW_INACTIVE;
        state Inactive_Out_Of_Neutral
          en:
          state_AuxEnable = INACTIVE;
          aux_Flow = AUX_FLOW_INACTIVE;
        (default) -> Aux_Enable_State.EMEA_CONTROLS.Functional.Inactive
        Aux_Enable_State.EMEA_CONTROLS.Functional.Inactive_Fault -> Aux_Enable_State.EMEA_CONTROLS.Functional.Enabled.Off : [state_RightPot != OORH...
            |  && state_RightPot != OORL...
            |  && state_System == SYS_NORMAL]
        Aux_Enable_State.EMEA_CONTROLS.Functional.Inactive -> Aux_Enable_State.EMEA_CONTROLS.Functional.Enabled : [state_Engine != ENGINE_CRANKING...
            | && state_System == SYS_NORMAL...
            | && state_Console == ACTIVE...
            | && flag_InitEERead]
        (junction 2294) -> Aux_Enable_State.EMEA_CONTROLS.Functional.Enabled.Off [order 2]
        Aux_Enable_State.EMEA_CONTROLS.Functional.Enabled -> Aux_Enable_State.EMEA_CONTROLS.Functional.Inactive_Fault [order 2] : [state_RightPot == OORH...
            |  || state_RightPot == OORL]
        Aux_Enable_State.EMEA_CONTROLS.Functional.Enabled -> Aux_Enable_State.EMEA_CONTROLS.Functional.Inactive [order 1] : [state_Engine == ENGINE_CRANKING...
            |  || state_System != SYS_NORMAL...
            |  || state_Console != ACTIVE]
        Aux_Enable_State.EMEA_CONTROLS.Functional.Inactive_Out_Of_Neutral -> Aux_Enable_State.EMEA_CONTROLS.Functional.Inactive_Fault [order 2] : [state_RightPot == OORH...
            |  || state_RightPot == OORL]
        Aux_Enable_State.EMEA_CONTROLS.Functional.Enabled -> Aux_Enable_State.EMEA_CONTROLS.Functional.Inactive_Out_Of_Neutral [order 3] : [state_RightPot == NOT_IN_NEUTRAL]
        (junction 2294) -> Aux_Enable_State.EMEA_CONTROLS.Functional.Enabled.Active.Standard_Flow [order 1] : [!aux_Inhibit...
            | &&(aux_DebounceButton || aux_DetentHold)]
            | {state_Buzzer = SINGLE_SHORT_BEEP;}
        Aux_Enable_State.EMEA_CONTROLS.Functional.Inactive_Out_Of_Neutral -> (junction 2294) [order 3] : [state_RightPot != NOT_IN_NEUTRAL]
        Aux_Enable_State.EMEA_CONTROLS.Functional.Inactive_Out_Of_Neutral -> Aux_Enable_State.EMEA_CONTROLS.Functional.Inactive [order 1] : [state_Console != ACTIVE]
      state Aux_Enable_State_ST
        state Inactive_Latched
          en:
          state_AuxEnable = INACTIVE;
        state Inactive_Temp
          en:
          state_AuxEnable = INACTIVE;
        state Standard_Flow
          en:
          aux_Flow = AUX_FLOW_STANDARD;
          state_AuxEnable = ACTIVE_MODE_ONE;
          ee_AuxFlow = AUX_FLOW_INACTIVE;
          matlab_UpdateEEVar();
        (default) -> Aux_Enable_State.EMEA_CONTROLS.Aux_Enable_State_ST.Standard_Flow
        Aux_Enable_State.EMEA_CONTROLS.Aux_Enable_State_ST.Standard_Flow -> Aux_Enable_State.EMEA_CONTROLS.Aux_Enable_State_ST.Inactive_Temp [order 1] : [state_Engine == ENGINE_CRANKING]
        Aux_Enable_State.EMEA_CONTROLS.Aux_Enable_State_ST.Standard_Flow -> Aux_Enable_State.EMEA_CONTROLS.Aux_Enable_State_ST.Inactive_Latched [order 2] : [state_AuxPot == OORH...
            |  || state_AuxPot == OORL...
            |  || state_Console == OORL...
            |  || state_Console == OORH...
            |  || state_AuxPot == NOT_IN_NEUTRAL...
            |  || state_System != SYS_NORMAL]
        Aux_Enable_State.EMEA_CONTROLS.Aux_Enable_State_ST.Inactive_Temp -> Aux_Enable_State.EMEA_CONTROLS.Aux_Enable_State_ST.Standard_Flow : [state_Engine != ENGINE_CRANKING]
        Aux_Enable_State.EMEA_CONTROLS.Aux_Enable_State_ST.Standard_Flow -> Aux_Enable_State.EMEA_CONTROLS.Aux_Enable_State_ST.Inactive_Latched [order 3] : disable_aux
      state Aux_Enable_State_ACD
        state Inactive_Latched
          en:
          state_AuxEnable = INACTIVE;
        state Inactive_Temp
          en:
          state_AuxEnable = INACTIVE;
        state Standard_Flow
          en:
          aux_Flow = AUX_FLOW_STANDARD;
          state_AuxEnable = ACTIVE_MODE_ONE;
        (default) -> Aux_Enable_State.EMEA_CONTROLS.Aux_Enable_State_ACD.Standard_Flow
        Aux_Enable_State.EMEA_CONTROLS.Aux_Enable_State_ACD.Standard_Flow -> Aux_Enable_State.EMEA_CONTROLS.Aux_Enable_State_ACD.Inactive_Temp [order 1] : [state_Engine == ENGINE_CRANKING]
        Aux_Enable_State.EMEA_CONTROLS.Aux_Enable_State_ACD.Standard_Flow -> Aux_Enable_State.EMEA_CONTROLS.Aux_Enable_State_ACD.Inactive_Latched [order 2] : [state_AuxPot == OORH...
            |  || state_AuxPot == OORL...
            |  || state_Console == OORL...
            |  || state_Console == OORH...
            |  || state_AuxPot == NOT_IN_NEUTRAL...
            |  || state_System != SYS_NORMAL]
        Aux_Enable_State.EMEA_CONTROLS.Aux_Enable_State_ACD.Standard_Flow -> Aux_Enable_State.EMEA_CONTROLS.Aux_Enable_State_ACD.Inactive_Latched [order 3] : disable_aux
        Aux_Enable_State.EMEA_CONTROLS.Aux_Enable_State_ACD.Inactive_Temp -> Aux_Enable_State.EMEA_CONTROLS.Aux_Enable_State_ACD.Standard_Flow : [state_Engine != ENGINE_CRANKING]
      (default) -> Aux_Enable_State.EMEA_CONTROLS.Functional
      Aux_Enable_State.EMEA_CONTROLS.Functional -> Aux_Enable_State.EMEA_CONTROLS.Aux_Enable_State_ST [order 1] : [(can_AuxEnable_ST == ACTIVE)...
          | && (state_ST_CAN == NORMAL)...
          | && (CD_STAUX_FUNCTION != NON_FUNCTIONAL)]
      Aux_Enable_State.EMEA_CONTROLS.Aux_Enable_State_ST -> Aux_Enable_State.EMEA_CONTROLS.Functional : [(can_AuxEnable_ST == INACTIVE)...
          | || (state_ST_CAN != NORMAL)]
      Aux_Enable_State.EMEA_CONTROLS.Functional -> Aux_Enable_State.EMEA_CONTROLS.Aux_Enable_State_ACD [order 2] : [(can_AuxEnable_ACD == ACTIVE)...
          | && (state_ACD_CAN == NORMAL)...
          | && (CD_RACD_FUNCTION != NON_FUNCTIONAL)]
      Aux_Enable_State.EMEA_CONTROLS.Aux_Enable_State_ACD -> Aux_Enable_State.EMEA_CONTROLS.Functional : [(can_AuxEnable_ACD == INACTIVE)...
          | || (state_ACD_CAN != NORMAL)]
    (default) -> Aux_Enable_State.Non_Functional
    Aux_Enable_State.Non_Functional -> PHOENIX_K2 [order 2] : [CD_AUX_FUNCTION == AUX_PHOENIX_K2 ]
    Aux_Enable_State.Non_Functional -> (junction 2103) [order 3] : [CD_AUX_FUNCTION == AUX_SIRIUS2]
    (junction 2103) -> SIRIUS2 [order 1] : [CD_AUX_HIGH_FLOW == NON_FUNCTIONAL]
    (junction 2103) -> SIRIUS2_HIFLOW [order 2]
    Aux_Enable_State.Non_Functional -> Aux_Enable_State.EMEA_CONTROLS [order 1] : [CD_AUX_FUNCTION == AUX_EMEA_CONTROLS]
  state Aux_Reset [parallel]
    en:
    state_ACD_old = state_ACD_CAN;
    state_ST_old = state_ST_CAN;
    settings: executionOrder=3
    Aux_Reset -> (junction 2342)
    (junction 2342) -> (junction 2343) [order 1] : [(CD_RACD_FUNCTION != NON_FUNCTIONAL)...
        | &&(state_ACD_CAN != state_ACD_old)]
    (junction 2342) -> (junction 2344) [order 2]
    (junction 2343) -> (junction 2345) : {
        | send(disable_detent, Detent_State);
        | state_ACD_old = state_ACD_CAN;
        | }
    (junction 2345) -> (junction 2344)
    (junction 2344) -> (junction 2346)
    (junction 2346) -> (junction 2347) [order 1] : [(CD_STAUX_FUNCTION != NON_FUNCTIONAL)...
        | &&(state_ST_CAN != state_ST_old)]
    (junction 2346) -> (junction 2348) [order 2]
    (junction 2347) -> (junction 2349) : {
        | send(disable_detent, Detent_State);
        | state_ST_old = state_ST_CAN;
        | }
    (junction 2349) -> (junction 2348)
    (junction 2348) -> (junction 2350)
  data: DetentRisingEdge input uint8; state_AuxEnable output uint8; ACTIVE_MODE_ONE parameter uint8; BASE_ACTIVE parameter uint8; BASE_DETENT parameter uint8; ENGINE_CRANKING parameter uint8; state_Detent output uint8; NON_FUNCTIONAL parameter uint8; INACTIVE parameter uint8; NO_DETENT parameter uint8; ROD_ACTIVE parameter uint8; ROD_DETENT parameter uint8; ACTIVE parameter uint8; state_AuxPot input uint8; state_Engine input uint8; state_Console input uint8; NOT_IN_NEUTRAL parameter uint8; OORH parameter uint8; OORL parameter uint8; aux_DebounceButton input uint8; state_System input uint8; aux_Flow output uint8; ee_AuxFlow data_store_memory uint8; AUX_FLOW_LOW parameter uint8; AUX_FLOW_MEDIUM parameter uint8; AUX_FLOW_HIGH parameter uint8; CD_AUX_ADJ_FLOW_FUNCTION parameter uint8; state_Buzzer data_store_memory uint8; SINGLE_SHORT_BEEP parameter uint8; CD_AUX_FUNCTION parameter uint8; state_DetentEnable output uint8; ERR_DETENT parameter uint8; aux_DetentHold input uint8; state_Buttons input uint8; NORMAL parameter uint8; SYS_NORMAL parameter uint8; DOUBLE_SHORT_BEEP parameter uint8; flag_InitEERead input boolean; can_AuxEnable_ST input uint8; can_AuxEnable_ACD input uint8; CD_STAUX_FUNCTION parameter uint8; CD_RACD_FUNCTION parameter uint8; state_ST_CAN input uint8; state_ACD_CAN input uint8; state_ACD_old local uint8; state_ST_old local uint8; button_AuxReleaseAck input boolean; button_AuxReleaseBack input boolean; button_AuxReleaseExit input boolean; CD_AUX_RELEASE_TIME parameter uint16; CD_AUX_CONFIRM_TIME parameter uint16; AUX_RELEASE_WAIT parameter uint8; AUX_RELEASE_ACK parameter uint8; AUX_RELEASE_IN_PROGRESS parameter uint8; AUX_RELEASE_CONFIRM parameter uint8; CD_AUX_RELEASE_FUNCTION parameter uint8; cmd_AuxRelease output uint8; ENGINE_NOT_RUNNING parameter uint8; ee_DeluxeG5 data_store_memory uint8; ENGINE_RUNNING parameter uint8; button_Aux input uint8; CD_AUX_DETENT_FLASH_TIME parameter uint16; ENGINE_INITIALIZE parameter uint8; AUX_SIRIUS2 parameter uint8; CD_AUX_HIGH_FLOW parameter uint8; AUX_FLOW_STANDARD parameter uint8; CD_DELUXE_GEN5_5_FUNCTION parameter uint8; state_AuxRelease output uint8; AUX_FLOW_INACTIVE parameter uint8; AUX_EMEA_CONTROLS parameter uint8; AUX_PHOENIX_K2 parameter uint8; state_RightPot input uint8; change_OffsetSelectSw input boolean; mode_RightControl input uint8; MODE_RIGHT_AUX1 parameter uint8; aux_Inhibit input boolean; state_EngineSpeed input uint8; NO_SIGNAL parameter uint8; previouslyActive local boolean; CD_EMEA_CONTROLS_OPTION_B parameter uint8; CD_EMEA_CONTROLS_OPTION_C parameter uint8; CD_RIGHT_POT_FUNCTION parameter uint8
  events: disable_aux local EITHER_EDGE_EVENT; disable_detent local EITHER_EDGE_EVENT
